simulink model "CCMSensor"  (R2022b)
settings: Solver=FixedStepDiscrete  StartTime=0.0  StopTime=10.0  FixedStep=0.01  RelTol=1e-3
undefined symbols (not defined in the file or in its folder): DioChannelType, IcuChannelType, P_DioLevelType
(part 2 of 2)

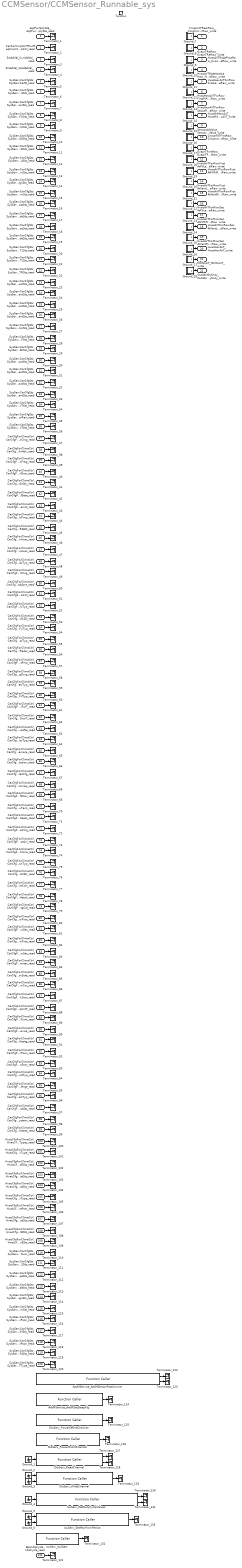
== system CCMSensor/CCMSensor_Runnable_sys ==
  AqsPwrSplySta_AqsPwrSplySta_read : Inport
  FanForCmptmtTRunReqFromClimaCtrl_FanForCmptmtTRunReqFromClimaCtrl_read : Inport  Port=2
  SrsAdVal_CurrAdVal_read : Inport  Port=3
  SrsAdVal_ValidAdVal_read : Inport  Port=4
  SysServCarCfgSts_SysServCarID_read : Inport  Port=5
  SysServCarCfgSts_SysServCCPAQSSts_read : Inport  Port=6
  SysServCarCfgSts_SysServCCPHEXSensorSts_read : Inport  Port=7
  SysServCarCfgSts_SysServCCPLin2AFUSts_read : Inport  Port=8
  SysServCarCfgSts_SysServCCPLin2AIUSts_read : Inport  Port=9
  SysServCarCfgSts_SysServCCPLin2CDSSts_read : Inport  Port=10
  SysServCarCfgSts_SysServCCPLin2PMSISts_read : Inport  Port=11
  SysServCarCfgSts_SysServCCPLin3VGASts_read : Inport  Port=12
  SysServCarCfgSts_SysServDUAirVentSnrSts_read : Inport  Port=13
  SysServCarCfgSts_SysServDUBlowerPwmSignSts_read : Inport  Port=14
  SysServCarCfgSts_SysServDUBlowerPwmSts_read : Inport  Port=15
  SysServCarCfgSts_SysServDUDCDefrostSts_read : Inport  Port=16
  SysServCarCfgSts_SysServDUDCModeSts_read : Inport  Port=17
  SysServCarCfgSts_SysServDUDCRecSts_read : Inport  Port=18
  SysServCarCfgSts_SysServDUDCSModeSts_read : Inport  Port=19
  SysServCarCfgSts_SysServDUDCSTLSts_read : Inport  Port=20
  SysServCarCfgSts_SysServDUDCTLSts_read : Inport  Port=21
  SysServCarCfgSts_SysServDUDCTRSts_read : Inport  Port=22
  SysServCarCfgSts_SysServDUFLFootSts_read : Inport  Port=23
  SysServCarCfgSts_SysServDUFLVentSts_read : Inport  Port=24
  SysServCarCfgSts_SysServDUFRFootSts_read : Inport  Port=25
  SysServCarCfgSts_SysServDUFRVentSts_read : Inport  Port=26
  SysServCarCfgSts_SysServDUIncarMotorSts_read : Inport  Port=27
  SysServCarCfgSts_SysServDULin1HBMFSts_read : Inport  Port=28
  SysServCarCfgSts_SysServDULin4HFAFSts_read : Inport  Port=29
  SysServCarCfgSts_SysServDUSFLFootSts_read : Inport  Port=30
  SysServCarCfgSts_SysServDUSFLVentSts_read : Inport  Port=31
  SysServCarCfgSts_SysServDUSFRFootSts_read : Inport  Port=32
  SysServCarCfgSts_SysServDUSFRVentSts_read : Inport  Port=33
  SysServCarCfgSts_SysServDUSnsrForFCmptmtTSts_read : Inport  Port=34
  SysServCarCfgSts_SysServDUSnsrForRair_read : Inport  Port=35
  SysServCarCfgSts_SysServDUSnsrForRCmptmtTSts_read : Inport  Port=36
  CarCfgForClimaCtrl_CarCfgForAirClng_read : Inport  Port=37
  CarCfgForClimaCtrl_CarCfgForAirClnrAdv_read : Inport  Port=38
  CarCfgForClimaCtrl_CarCfgForAirFrag_read : Inport  Port=39
  CarCfgForClimaCtrl_CarCfgForAirMtrlSnsr_read : Inport  Port=40
  CarCfgForClimaCtrl_CarCfgForAirQly_read : Inport  Port=41
  CarCfgForClimaCtrl_CarCfgForAmntOfSeat_read : Inport  Port=42
  CarCfgForClimaCtrl_CarCfgForAutDemst_read : Inport  Port=43
  CarCfgForClimaCtrl_CarCfgForAuxWtrPmp_read : Inport  Port=44
  CarCfgForClimaCtrl_CarCfgForBRAND_read : Inport  Port=45
  CarCfgForClimaCtrl_CarCfgForBypVlvInHvac_read : Inport  Port=46
  CarCfgForClimaCtrl_CarCfgForClsVlvInHvac_read : Inport  Port=47
  CarCfgForClimaCtrl_CarCfgForCmprAcTyp_read : Inport  Port=48
  CarCfgForClimaCtrl_CarCfgForCmptmtClng_read : Inport  Port=49
  CarCfgForClimaCtrl_CarCfgForElecVentAdjmt_read : Inport  Port=50
  CarCfgForClimaCtrl_CarCfgForExtVentnClimaCtrl_read : Inport  Port=51
  CarCfgForClimaCtrl_CarCfgForFlcTyp_read : Inport  Port=52
  CarCfgForClimaCtrl_CarCfgForFoldCSD_read : Inport  Port=53
  CarCfgForClimaCtrl_CarCfgForFuTyp_read : Inport  Port=54
  CarCfgForClimaCtrl_CarCfgForGlassTyp_read : Inport  Port=55
  CarCfgForClimaCtrl_CarCfgForHeadRadar_read : Inport  Port=56
  CarCfgForClimaCtrl_CarCfgForHeatPmp_read : Inport  Port=57
  CarCfgForClimaCtrl_CarCfgForHeatrDurgDrvg_read : Inport  Port=58
  CarCfgForClimaCtrl_CarCfgForHeatrTyp_read : Inport  Port=59
  CarCfgForClimaCtrl_CarCfgForHvacFilTyp_read : Inport  Port=60
  CarCfgForClimaCtrl_CarCfgForHvacFlrSnsrForT_read : Inport  Port=61
  CarCfgForClimaCtrl_CarCfgForHvacHeatrSnsrT_read : Inport  Port=62
  CarCfgForClimaCtrl_CarCfgForHvacRe_read : Inport  Port=63
  CarCfgForClimaCtrl_CarCfgForHvacTyp_read : Inport  Port=64
  CarCfgForClimaCtrl_CarCfgForInteriorViewCamera_read : Inport  Port=65
  CarCfgForClimaCtrl_CarCfgForLifeDetection_read : Inport  Port=66
  CarCfgForClimaCtrl_CarCfgForLoVoltElecCfg_read : Inport  Port=67
  CarCfgForClimaCtrl_CarCfgForNavSysandIntnmap_read : Inport  Port=68
  CarCfgForClimaCtrl_CarCfgForNrOfDoor_read : Inport  Port=69
  CarCfgForClimaCtrl_CarCfgForPipeInFuTank_read : Inport  Port=70
  CarCfgForClimaCtrl_CarCfgForPowerSeats_read : Inport  Port=71
  CarCfgForClimaCtrl_CarCfgForPreClng_read : Inport  Port=72
  CarCfgForClimaCtrl_CarCfgForPrkgCDSNotAvl_read : Inport  Port=73
  CarCfgForClimaCtrl_CarCfgForPrkgClima_read : Inport  Port=74
  CarCfgForClimaCtrl_CarCfgForPrpsnTyp_read : Inport  Port=75
  CarCfgForClimaCtrl_CarCfgForPTCInFrontHVAC_read : Inport  Port=76
  CarCfgForClimaCtrl_CarCfgForRemEngStrtPreCdn_read : Inport  Port=77
  CarCfgForClimaCtrl_CarCfgForRoofHatch_read : Inport  Port=78
  CarCfgForClimaCtrl_CarCfgForSeatHeatgCal_read : Inport  Port=79
  CarCfgForClimaCtrl_CarCfgForSeatHeatgForRowFirst_read : Inport  Port=80
  CarCfgForClimaCtrl_CarCfgForSeatHeatgForRowSec_read : Inport  Port=81
  CarCfgForClimaCtrl_CarCfgForSeatVentnForRowFirst_read : Inport  Port=82
  CarCfgForClimaCtrl_CarCfgForSeatVentnForRowSec_read : Inport  Port=83
  CarCfgForClimaCtrl_CarCfgForSecRowVentnCenter_read : Inport  Port=84
  CarCfgForClimaCtrl_CarCfgForSecRowVentnSide_read : Inport  Port=85
  CarCfgForClimaCtrl_CarCfgForSmallFlowCyc_read : Inport  Port=86
  CarCfgForClimaCtrl_CarCfgForSmkSnsr_read : Inport  Port=87
  CarCfgForClimaCtrl_CarCfgForSnsrForCmptmtT_read : Inport  Port=88
  CarCfgForClimaCtrl_CarCfgForSnsrHumPrsnt_read : Inport  Port=89
  CarCfgForClimaCtrl_CarCfgForStartDevice_read : Inport  Port=90
  CarCfgForClimaCtrl_CarCfgForSteerWhlHeatg_read : Inport  Port=91
  CarCfgForClimaCtrl_CarCfgForSteerWhlPosn_read : Inport  Port=92
  CarCfgForClimaCtrl_CarCfgForSunSnsr_read : Inport  Port=93
  CarCfgForClimaCtrl_CarCfgForTelmNodTyp_read : Inport  Port=94
  CarCfgForClimaCtrl_CarCfgForThermMngt_read : Inport  Port=95
  CarCfgForClimaCtrl_CarCfgForVehTyp_read : Inport  Port=96
  CarCfgForClimaCtrl_CarCfgForVgaMotorExistSts_read : Inport  Port=97
  CarCfgForClimaCtrl_CarCfgForWadingAssistSystem_read : Inport  Port=98
  CarCfgForClimaCtrl_CarCfgForWindHeatd_read : Inport  Port=99
  HvacCfgForClimaCtrl_HvacCfgForAQSTypes_read : Inport  Port=100
  HvacCfgForClimaCtrl_HvacCfgForHvacFrontBlowerType_read : Inport  Port=101
  HvacCfgForClimaCtrl_HvacCfgForHvacLowSideRETXVESts_read : Inport  Port=102
  HvacCfgForClimaCtrl_HvacCfgForHvacMiscExistSts_read : Inport  Port=103
  HvacCfgForClimaCtrl_HvacCfgForHvacMotorExistSts_read : Inport  Port=104
  HvacCfgForClimaCtrl_HvacCfgForHvacReBlowerType_read : Inport  Port=105
  HvacCfgForClimaCtrl_HvacCfgForHvacReVentPstn_read : Inport  Port=106
  HvacCfgForClimaCtrl_HvacCfgForHvacSensorExistSts_read : Inport  Port=107
  HvacCfgForClimaCtrl_HvacCfgForHvacSnsrRCmptmtESts_read : Inport  Port=108
  HvacCfgForClimaCtrl_HvacCfgForHvacSnsrReHexESts_read : Inport  Port=109
  SysServCarCfgSts_SysServCCPBGMNum_read : Inport  Port=110
  SysServCarCfgSts_SysServCCPLin2AQSSts_read : Inport  Port=111
  SysServCarCfgSts_SysServDUBlowerPwmSignTypeSts_read : Inport  Port=112
  SysServCarCfgSts_SysServDUDCFLMdeSts_read : Inport  Port=113
  SysServCarCfgSts_SysServDUReBlowerPwmSignSts_read : Inport  Port=114
  SysServCarCfgSts_SysServDUReBlowerPwmSts_read : Inport  Port=115
  SysServCarCfgSts_SysServFrntHEXSnsrPosn_read : Inport  Port=116
  SysServCarCfgSts_SysServLowSideRETXVSts_read : Inport  Port=117
  SysServCarCfgSts_SysServReHEXSnsrPosn_read : Inport  Port=118
  SysServCarCfgSts_SysServRHFASts_read : Inport  Port=119
  SysServCarCfgSts_SysServSnsrForFCmptmtTType_read : Inport  Port=120
  BswLifeCycle_LifeCycle_read : Inport  Port=121
  function : TriggerPort  TriggerType=function-call  VariantControl=(inherit)  SampleTimeType=periodic  SampleTime=-1
  AdcMService_AdcMSensorFastConver : FunctionCaller  FunctionPrototype=[SensorFastConverFlag,ERR] = AdcMService_AdcMSensorFastConver()  OutputArgumentSpecifications=P_AdcMSensorFastConver, P_Std_ReturnType
  AdcMService_AdcMSetSleepFlg : FunctionCaller  FunctionPrototype=ERR = AdcMService_AdcMSetSleepFlg()  OutputArgumentSpecifications=P_Std_ReturnType
  DioServ_PowerOffInitChannel : FunctionCaller  FunctionPrototype=ERR = DioServ_PowerOffInitChannel()  OutputArgumentSpecifications=P_Std_ReturnType
  DioServ_PowerOnInitChannel : FunctionCaller  FunctionPrototype=ERR = DioServ_PowerOnInitChannel()  OutputArgumentSpecifications=P_Std_ReturnType
  DioServ_ReadChannel : FunctionCaller  FunctionPrototype=[Level,ERR] = DioServ_ReadChannel(ChannelId)  InputArgumentSpecifications=DioChannelType(0)  OutputArgumentSpecifications=P_DioLevelType, P_Std_ReturnType
  DioServ_WriteChannel : FunctionCaller  FunctionPrototype=ERR = DioServ_WriteChannel(ChannelId,Level)  InputArgumentSpecifications=DioChannelType(0), P_DioLevelType  OutputArgumentSpecifications=P_Std_ReturnType
  Ground_{1..6} (6 alike) : Ground
  Ground_10 : Subsystem
  Ground_11 : Subsystem
  Ground_12 : Subsystem
  Ground_13 : Subsystem
  Ground_14 : Subsystem
  Ground_15 : Subsystem
  Ground_16 : Subsystem
  Ground_17 : Subsystem
  Ground_18 : Subsystem
  Ground_19 : Subsystem
  Ground_20 : Subsystem
  Ground_21 : Subsystem
  Ground_22 : Subsystem
  Ground_23 : Subsystem
  Ground_24 : Subsystem
  Ground_25 : Subsystem
  Ground_26 : Subsystem
  Ground_27 : Subsystem
  Ground_28 : Subsystem
  Ground_7 : Subsystem
  Ground_8 : Subsystem
  Ground_9 : Subsystem
  IcuServ_GetDutyCycleValues : FunctionCaller  FunctionPrototype=[DutyCycleValues,ERR] = IcuServ_GetDutyCycleValues(Channel)  InputArgumentSpecifications=IcuChannelType(0)  OutputArgumentSpecifications=P_IcuDutyCycleType, P_Std_ReturnType
  IcuServ_IcuStart : FunctionCaller  FunctionPrototype=ERR = IcuServ_IcuStart()  OutputArgumentSpecifications=P_Std_ReturnType
  IcuServ_SetMaxPwmPeriod : FunctionCaller  FunctionPrototype=ERR = IcuServ_SetMaxPwmPeriod(Channel,MaxPwmPeriod)  InputArgumentSpecifications=IcuChannelType(0), uint16(1)  OutputArgumentSpecifications=P_Std_ReturnType
  Terminator_{1..133} (133 alike) : Terminator
  CmptmtTRearRaw_CmptmtTRearRaw_write : Outport
      settings: EnsureOutportIsVirtual=on
  EvaprTReRaw_EvaprTReRaw_write : Outport  Port=2
      settings: EnsureOutportIsVirtual=on
  HvacAirTReAtFlrLeRaw_HvacAirTReAtFlrLeRaw_write : Outport  Port=3
      settings: EnsureOutportIsVirtual=on
  HvacAirTReAtVentLeRaw_HvacAirTReAtVentLeRaw_write : Outport  Port=4
      settings: EnsureOutportIsVirtual=on
  HvacHexAirTForRowFirstLeRaw_HvacHexAirTForRowFirstLeRaw_write : Outport  Port=5
      settings: EnsureOutportIsVirtual=on
  HvacHexAirTForRowFirstRiRaw_HvacHexAirTForRowFirstRiRaw_write : Outport  Port=6
      settings: EnsureOutportIsVirtual=on
  HvacHexAirTForRowSecLeRaw_HvacHexAirTForRowSecLeRaw_write : Outport  Port=7
      settings: EnsureOutportIsVirtual=on
  HvacReHexAirT_HvacReHexAirT_write : Outport  Port=8
      settings: EnsureOutportIsVirtual=on
  SensorAdValue_SensorAdValue_write : Outport  Port=9
      settings: EnsureOutportIsVirtual=on
  CmptmtTFrntRaw_CmptmtTFrntRaw_write : Outport  Port=10
      settings: EnsureOutportIsVirtual=on
  EvaprTFrntRaw_EvaprTFrntRaw_write : Outport  Port=11
      settings: EnsureOutportIsVirtual=on
  HvacAirTForRowFirstAtFlrLeRaw_HvacAirTForRowFirstAtFlrLeRaw_write : Outport  Port=12
      settings: EnsureOutportIsVirtual=on
  HvacAirTForRowFirstAtFlrRiRaw_HvacAirTForRowFirstAtFlrRiRaw_write : Outport  Port=13
      settings: EnsureOutportIsVirtual=on
  HvacAirTForRowFirstAtVentLeRaw_HvacAirTForRowFirstAtVentLeRaw_write : Outport  Port=14
      settings: EnsureOutportIsVirtual=on
  HvacAirTForRowFirstAtVentRiRaw_HvacAirTForRowFirstAtVentRiRaw_write : Outport  Port=15
      settings: EnsureOutportIsVirtual=on
  HvacAirTForRowSecAtFlrLeRaw_HvacAirTForRowSecAtFlrLeRaw_write : Outport  Port=16
      settings: EnsureOutportIsVirtual=on
  HvacAirTForRowSecAtFlrRiRaw_HvacAirTForRowSecAtFlrRiRaw_write : Outport  Port=17
      settings: EnsureOutportIsVirtual=on
  HvacAirTForRowSecAtVentLeRaw_HvacAirTForRowSecAtVentLeRaw_write : Outport  Port=18
      settings: EnsureOutportIsVirtual=on
  HvacAirTForRowSecAtVentRiRaw_HvacAirTForRowSecAtVentRiRaw_write : Outport  Port=19
      settings: EnsureOutportIsVirtual=on
  HvacHexAirT_HvacHexAirT_write : Outport  Port=20
      settings: EnsureOutportIsVirtual=on
  MtrlSnsrT_MtrlSnsrT_write : Outport  Port=21
      settings: EnsureOutportIsVirtual=on
  OutdAirQlyDuty_OutdAirQlyDuty_write : Outport  Port=22
      settings: EnsureOutportIsVirtual=on
  wires:
  AqsPwrSplySta_AqsPwrSplySta_read/1 -> Terminator_1/1
  FanForCmptmtTRunReqFromClimaCtrl_FanForCmptmtTRunReqFromClimaCtrl_read/1 -> Terminator_2/1
  SrsAdVal_CurrAdVal_read/1 -> Terminator_3/1
  SrsAdVal_ValidAdVal_read/1 -> Terminator_4/1
  SysServCarCfgSts_SysServCarID_read/1 -> Terminator_5/1
  SysServCarCfgSts_SysServCCPAQSSts_read/1 -> Terminator_6/1
  SysServCarCfgSts_SysServCCPHEXSensorSts_read/1 -> Terminator_7/1
  SysServCarCfgSts_SysServCCPLin2AFUSts_read/1 -> Terminator_8/1
  SysServCarCfgSts_SysServCCPLin2AIUSts_read/1 -> Terminator_9/1
  SysServCarCfgSts_SysServCCPLin2CDSSts_read/1 -> Terminator_10/1
  SysServCarCfgSts_SysServCCPLin2PMSISts_read/1 -> Terminator_11/1
  SysServCarCfgSts_SysServCCPLin3VGASts_read/1 -> Terminator_12/1
  SysServCarCfgSts_SysServDUAirVentSnrSts_read/1 -> Terminator_13/1
  SysServCarCfgSts_SysServDUBlowerPwmSignSts_read/1 -> Terminator_14/1
  SysServCarCfgSts_SysServDUBlowerPwmSts_read/1 -> Terminator_15/1
  SysServCarCfgSts_SysServDUDCDefrostSts_read/1 -> Terminator_16/1
  SysServCarCfgSts_SysServDUDCModeSts_read/1 -> Terminator_17/1
  SysServCarCfgSts_SysServDUDCRecSts_read/1 -> Terminator_18/1
  SysServCarCfgSts_SysServDUDCSModeSts_read/1 -> Terminator_19/1
  SysServCarCfgSts_SysServDUDCSTLSts_read/1 -> Terminator_20/1
  SysServCarCfgSts_SysServDUDCTLSts_read/1 -> Terminator_21/1
  SysServCarCfgSts_SysServDUDCTRSts_read/1 -> Terminator_22/1
  SysServCarCfgSts_SysServDUFLFootSts_read/1 -> Terminator_23/1
  SysServCarCfgSts_SysServDUFLVentSts_read/1 -> Terminator_24/1
  SysServCarCfgSts_SysServDUFRFootSts_read/1 -> Terminator_25/1
  SysServCarCfgSts_SysServDUFRVentSts_read/1 -> Terminator_26/1
  SysServCarCfgSts_SysServDUIncarMotorSts_read/1 -> Terminator_27/1
  SysServCarCfgSts_SysServDULin1HBMFSts_read/1 -> Terminator_28/1
  SysServCarCfgSts_SysServDULin4HFAFSts_read/1 -> Terminator_29/1
  SysServCarCfgSts_SysServDUSFLFootSts_read/1 -> Terminator_30/1
  SysServCarCfgSts_SysServDUSFLVentSts_read/1 -> Terminator_31/1
  SysServCarCfgSts_SysServDUSFRFootSts_read/1 -> Terminator_32/1
  SysServCarCfgSts_SysServDUSFRVentSts_read/1 -> Terminator_33/1
  SysServCarCfgSts_SysServDUSnsrForFCmptmtTSts_read/1 -> Terminator_34/1
  SysServCarCfgSts_SysServDUSnsrForRair_read/1 -> Terminator_35/1
  SysServCarCfgSts_SysServDUSnsrForRCmptmtTSts_read/1 -> Terminator_36/1
  CarCfgForClimaCtrl_CarCfgForAirClng_read/1 -> Terminator_37/1
  CarCfgForClimaCtrl_CarCfgForAirClnrAdv_read/1 -> Terminator_38/1
  CarCfgForClimaCtrl_CarCfgForAirFrag_read/1 -> Terminator_39/1
  CarCfgForClimaCtrl_CarCfgForAirMtrlSnsr_read/1 -> Terminator_40/1
  CarCfgForClimaCtrl_CarCfgForAirQly_read/1 -> Terminator_41/1
  CarCfgForClimaCtrl_CarCfgForAmntOfSeat_read/1 -> Terminator_42/1
  CarCfgForClimaCtrl_CarCfgForAutDemst_read/1 -> Terminator_43/1
  CarCfgForClimaCtrl_CarCfgForAuxWtrPmp_read/1 -> Terminator_44/1
  CarCfgForClimaCtrl_CarCfgForBRAND_read/1 -> Terminator_45/1
  CarCfgForClimaCtrl_CarCfgForBypVlvInHvac_read/1 -> Terminator_46/1
  CarCfgForClimaCtrl_CarCfgForClsVlvInHvac_read/1 -> Terminator_47/1
  CarCfgForClimaCtrl_CarCfgForCmprAcTyp_read/1 -> Terminator_48/1
  CarCfgForClimaCtrl_CarCfgForCmptmtClng_read/1 -> Terminator_49/1
  CarCfgForClimaCtrl_CarCfgForElecVentAdjmt_read/1 -> Terminator_50/1
  CarCfgForClimaCtrl_CarCfgForExtVentnClimaCtrl_read/1 -> Terminator_51/1
  CarCfgForClimaCtrl_CarCfgForFlcTyp_read/1 -> Terminator_52/1
  CarCfgForClimaCtrl_CarCfgForFoldCSD_read/1 -> Terminator_53/1
  CarCfgForClimaCtrl_CarCfgForFuTyp_read/1 -> Terminator_54/1
  CarCfgForClimaCtrl_CarCfgForGlassTyp_read/1 -> Terminator_55/1
  CarCfgForClimaCtrl_CarCfgForHeadRadar_read/1 -> Terminator_56/1
  CarCfgForClimaCtrl_CarCfgForHeatPmp_read/1 -> Terminator_57/1
  CarCfgForClimaCtrl_CarCfgForHeatrDurgDrvg_read/1 -> Terminator_58/1
  CarCfgForClimaCtrl_CarCfgForHeatrTyp_read/1 -> Terminator_59/1
  CarCfgForClimaCtrl_CarCfgForHvacFilTyp_read/1 -> Terminator_60/1
  CarCfgForClimaCtrl_CarCfgForHvacFlrSnsrForT_read/1 -> Terminator_61/1
  CarCfgForClimaCtrl_CarCfgForHvacHeatrSnsrT_read/1 -> Terminator_62/1
  CarCfgForClimaCtrl_CarCfgForHvacRe_read/1 -> Terminator_63/1
  CarCfgForClimaCtrl_CarCfgForHvacTyp_read/1 -> Terminator_64/1
  CarCfgForClimaCtrl_CarCfgForInteriorViewCamera_read/1 -> Terminator_65/1
  CarCfgForClimaCtrl_CarCfgForLifeDetection_read/1 -> Terminator_66/1
  CarCfgForClimaCtrl_CarCfgForLoVoltElecCfg_read/1 -> Terminator_67/1
  CarCfgForClimaCtrl_CarCfgForNavSysandIntnmap_read/1 -> Terminator_68/1
  CarCfgForClimaCtrl_CarCfgForNrOfDoor_read/1 -> Terminator_69/1
  CarCfgForClimaCtrl_CarCfgForPipeInFuTank_read/1 -> Terminator_70/1
  CarCfgForClimaCtrl_CarCfgForPowerSeats_read/1 -> Terminator_71/1
  CarCfgForClimaCtrl_CarCfgForPreClng_read/1 -> Terminator_72/1
  CarCfgForClimaCtrl_CarCfgForPrkgCDSNotAvl_read/1 -> Terminator_73/1
  CarCfgForClimaCtrl_CarCfgForPrkgClima_read/1 -> Terminator_74/1
  CarCfgForClimaCtrl_CarCfgForPrpsnTyp_read/1 -> Terminator_75/1
  CarCfgForClimaCtrl_CarCfgForPTCInFrontHVAC_read/1 -> Terminator_76/1
  CarCfgForClimaCtrl_CarCfgForRemEngStrtPreCdn_read/1 -> Terminator_77/1
  CarCfgForClimaCtrl_CarCfgForRoofHatch_read/1 -> Terminator_78/1
  CarCfgForClimaCtrl_CarCfgForSeatHeatgCal_read/1 -> Terminator_79/1
  CarCfgForClimaCtrl_CarCfgForSeatHeatgForRowFirst_read/1 -> Terminator_80/1
  CarCfgForClimaCtrl_CarCfgForSeatHeatgForRowSec_read/1 -> Terminator_81/1
  CarCfgForClimaCtrl_CarCfgForSeatVentnForRowFirst_read/1 -> Terminator_82/1
  CarCfgForClimaCtrl_CarCfgForSeatVentnForRowSec_read/1 -> Terminator_83/1
  CarCfgForClimaCtrl_CarCfgForSecRowVentnCenter_read/1 -> Terminator_84/1
  CarCfgForClimaCtrl_CarCfgForSecRowVentnSide_read/1 -> Terminator_85/1
  CarCfgForClimaCtrl_CarCfgForSmallFlowCyc_read/1 -> Terminator_86/1
  CarCfgForClimaCtrl_CarCfgForSmkSnsr_read/1 -> Terminator_87/1
  CarCfgForClimaCtrl_CarCfgForSnsrForCmptmtT_read/1 -> Terminator_88/1
  CarCfgForClimaCtrl_CarCfgForSnsrHumPrsnt_read/1 -> Terminator_89/1
  CarCfgForClimaCtrl_CarCfgForStartDevice_read/1 -> Terminator_90/1
  CarCfgForClimaCtrl_CarCfgForSteerWhlHeatg_read/1 -> Terminator_91/1
  CarCfgForClimaCtrl_CarCfgForSteerWhlPosn_read/1 -> Terminator_92/1
  CarCfgForClimaCtrl_CarCfgForSunSnsr_read/1 -> Terminator_93/1
  CarCfgForClimaCtrl_CarCfgForTelmNodTyp_read/1 -> Terminator_94/1
  CarCfgForClimaCtrl_CarCfgForThermMngt_read/1 -> Terminator_95/1
  CarCfgForClimaCtrl_CarCfgForVehTyp_read/1 -> Terminator_96/1
  CarCfgForClimaCtrl_CarCfgForVgaMotorExistSts_read/1 -> Terminator_97/1
  CarCfgForClimaCtrl_CarCfgForWadingAssistSystem_read/1 -> Terminator_98/1
  CarCfgForClimaCtrl_CarCfgForWindHeatd_read/1 -> Terminator_99/1
  HvacCfgForClimaCtrl_HvacCfgForAQSTypes_read/1 -> Terminator_100/1
  HvacCfgForClimaCtrl_HvacCfgForHvacFrontBlowerType_read/1 -> Terminator_101/1
  HvacCfgForClimaCtrl_HvacCfgForHvacLowSideRETXVESts_read/1 -> Terminator_102/1
  HvacCfgForClimaCtrl_HvacCfgForHvacMiscExistSts_read/1 -> Terminator_103/1
  HvacCfgForClimaCtrl_HvacCfgForHvacMotorExistSts_read/1 -> Terminator_104/1
  HvacCfgForClimaCtrl_HvacCfgForHvacReBlowerType_read/1 -> Terminator_105/1
  HvacCfgForClimaCtrl_HvacCfgForHvacReVentPstn_read/1 -> Terminator_106/1
  HvacCfgForClimaCtrl_HvacCfgForHvacSensorExistSts_read/1 -> Terminator_107/1
  HvacCfgForClimaCtrl_HvacCfgForHvacSnsrRCmptmtESts_read/1 -> Terminator_108/1
  HvacCfgForClimaCtrl_HvacCfgForHvacSnsrReHexESts_read/1 -> Terminator_109/1
  SysServCarCfgSts_SysServCCPBGMNum_read/1 -> Terminator_110/1
  SysServCarCfgSts_SysServCCPLin2AQSSts_read/1 -> Terminator_111/1
  SysServCarCfgSts_SysServDUBlowerPwmSignTypeSts_read/1 -> Terminator_112/1
  SysServCarCfgSts_SysServDUDCFLMdeSts_read/1 -> Terminator_113/1
  SysServCarCfgSts_SysServDUReBlowerPwmSignSts_read/1 -> Terminator_114/1
  SysServCarCfgSts_SysServDUReBlowerPwmSts_read/1 -> Terminator_115/1
  SysServCarCfgSts_SysServFrntHEXSnsrPosn_read/1 -> Terminator_116/1
  SysServCarCfgSts_SysServLowSideRETXVSts_read/1 -> Terminator_117/1
  SysServCarCfgSts_SysServReHEXSnsrPosn_read/1 -> Terminator_118/1
  SysServCarCfgSts_SysServRHFASts_read/1 -> Terminator_119/1
  SysServCarCfgSts_SysServSnsrForFCmptmtTType_read/1 -> Terminator_120/1
  BswLifeCycle_LifeCycle_read/1 -> Terminator_121/1
  AdcMService_AdcMSensorFastConver/1 -> Terminator_122/1
  AdcMService_AdcMSensorFastConver/2 -> Terminator_123/1
  AdcMService_AdcMSetSleepFlg/1 -> Terminator_124/1
  DioServ_PowerOffInitChannel/1 -> Terminator_125/1
  DioServ_PowerOnInitChannel/1 -> Terminator_126/1
  Ground_1/1 -> DioServ_ReadChannel/1
  DioServ_ReadChannel/1 -> Terminator_127/1
  DioServ_ReadChannel/2 -> Terminator_128/1
  Ground_2/1 -> DioServ_WriteChannel/1
  Ground_3/1 -> DioServ_WriteChannel/2
  DioServ_WriteChannel/1 -> Terminator_129/1
  Ground_4/1 -> IcuServ_GetDutyCycleValues/1
  IcuServ_GetDutyCycleValues/1 -> Terminator_130/1
  IcuServ_GetDutyCycleValues/2 -> Terminator_131/1
  IcuServ_IcuStart/1 -> Terminator_132/1
  Ground_5/1 -> IcuServ_SetMaxPwmPeriod/1
  Ground_6/1 -> IcuServ_SetMaxPwmPeriod/2
  IcuServ_SetMaxPwmPeriod/1 -> Terminator_133/1
  Ground_7/1(Out1) -> CmptmtTRearRaw_CmptmtTRearRaw_write/1
  Ground_8/1(Out1) -> EvaprTReRaw_EvaprTReRaw_write/1
  Ground_9/1(Out1) -> HvacAirTReAtFlrLeRaw_HvacAirTReAtFlrLeRaw_write/1
  Ground_10/1(Out1) -> HvacAirTReAtVentLeRaw_HvacAirTReAtVentLeRaw_write/1
  Ground_11/1(Out1) -> HvacHexAirTForRowFirstLeRaw_HvacHexAirTForRowFirstLeRaw_write/1
  Ground_12/1(Out1) -> HvacHexAirTForRowFirstRiRaw_HvacHexAirTForRowFirstRiRaw_write/1
  Ground_13/1(Out1) -> HvacHexAirTForRowSecLeRaw_HvacHexAirTForRowSecLeRaw_write/1
  Ground_14/1(Out1) -> HvacReHexAirT_HvacReHexAirT_write/1
  Ground_15/1(Out1) -> SensorAdValue_SensorAdValue_write/1
  Ground_16/1(Out1) -> CmptmtTFrntRaw_CmptmtTFrntRaw_write/1
  Ground_17/1(Out1) -> EvaprTFrntRaw_EvaprTFrntRaw_write/1
  Ground_18/1(Out1) -> HvacAirTForRowFirstAtFlrLeRaw_HvacAirTForRowFirstAtFlrLeRaw_write/1
  Ground_19/1(Out1) -> HvacAirTForRowFirstAtFlrRiRaw_HvacAirTForRowFirstAtFlrRiRaw_write/1
  Ground_20/1(Out1) -> HvacAirTForRowFirstAtVentLeRaw_HvacAirTForRowFirstAtVentLeRaw_write/1
  Ground_21/1(Out1) -> HvacAirTForRowFirstAtVentRiRaw_HvacAirTForRowFirstAtVentRiRaw_write/1
  Ground_22/1(Out1) -> HvacAirTForRowSecAtFlrLeRaw_HvacAirTForRowSecAtFlrLeRaw_write/1
  Ground_23/1(Out1) -> HvacAirTForRowSecAtFlrRiRaw_HvacAirTForRowSecAtFlrRiRaw_write/1
  Ground_24/1(Out1) -> HvacAirTForRowSecAtVentLeRaw_HvacAirTForRowSecAtVentLeRaw_write/1
  Ground_25/1(Out1) -> HvacAirTForRowSecAtVentRiRaw_HvacAirTForRowSecAtVentRiRaw_write/1
  Ground_26/1(Out1) -> HvacHexAirT_HvacHexAirT_write/1
  Ground_27/1(Out1) -> MtrlSnsrT_MtrlSnsrT_write/1
  Ground_28/1(Out1) -> OutdAirQlyDuty_OutdAirQlyDuty_write/1

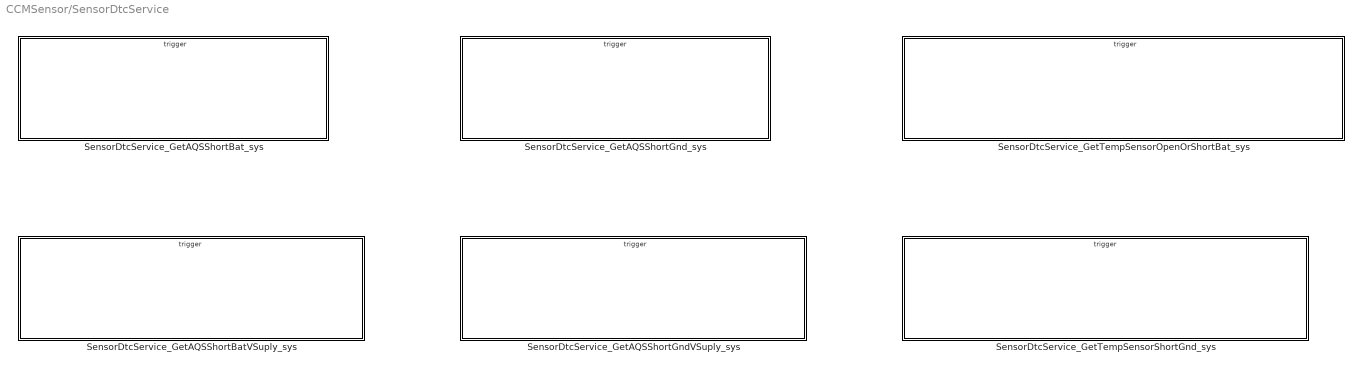
== system CCMSensor/SensorDtcService ==
  SensorDtcService_GetAQSShortBatVSuply_sys : Subsystem
  SensorDtcService_GetAQSShortBat_sys : Subsystem
  SensorDtcService_GetAQSShortGndVSuply_sys : Subsystem
  SensorDtcService_GetAQSShortGnd_sys : Subsystem
  SensorDtcService_GetTempSensorOpenOrShortBat_sys : Subsystem
  SensorDtcService_GetTempSensorShortGnd_sys : Subsystem

== system CCMSensor/SensorParaService ==
  SensorParaService_GetValidValue_sys : Subsystem

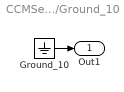
== system CCMSensor/CCMSensor_Runnable_sys/Ground_10 ==
  Ground_10 : Ground
  Out1 : Outport  OutDataTypeStr=Bus: HvacAirTReAtVentLe  PortDimensions=1
  wires:
  Ground_10/1 -> Out1/1

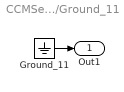
== system CCMSensor/CCMSensor_Runnable_sys/Ground_11 ==
  Ground_11 : Ground
  Out1 : Outport  OutDataTypeStr=Bus: HvacHexAirTForRowFirstLe  PortDimensions=1
  wires:
  Ground_11/1 -> Out1/1

== system CCMSensor/CCMSensor_Runnable_sys/Ground_12 ==
  Ground_12 : Ground
  Out1 : Outport  OutDataTypeStr=Bus: HvacHexAirTForRowFirstRi  PortDimensions=1
  wires:
  Ground_12/1 -> Out1/1

== system CCMSensor/CCMSensor_Runnable_sys/Ground_13 ==
  Ground_13 : Ground
  Out1 : Outport  OutDataTypeStr=Bus: HvacHexAirTForRowSecLe  PortDimensions=1
  wires:
  Ground_13/1 -> Out1/1

== system CCMSensor/CCMSensor_Runnable_sys/Ground_14 ==
  Ground_14 : Ground
  Out1 : Outport  OutDataTypeStr=Bus: HvacAirTForHeatrFrnt  PortDimensions=1
  wires:
  Ground_14/1 -> Out1/1

== system CCMSensor/CCMSensor_Runnable_sys/Ground_15 ==
  Ground_15 : Ground
  Out1 : Outport  OutDataTypeStr=Bus: SensorAd  PortDimensions=1
  wires:
  Ground_15/1 -> Out1/1

== system CCMSensor/CCMSensor_Runnable_sys/Ground_16 ==
  Ground_16 : Ground
  Out1 : Outport  OutDataTypeStr=Bus: CmptmtTFrnt  PortDimensions=1
  wires:
  Ground_16/1 -> Out1/1

== system CCMSensor/CCMSensor_Runnable_sys/Ground_17 ==
  Ground_17 : Ground
  Out1 : Outport  OutDataTypeStr=Bus: EvaprTFrnt  PortDimensions=1
  wires:
  Ground_17/1 -> Out1/1

== system CCMSensor/CCMSensor_Runnable_sys/Ground_18 ==
  Ground_18 : Ground
  Out1 : Outport  OutDataTypeStr=Bus: HvacAirTForRowFirstAtFlrLe  PortDimensions=1
  wires:
  Ground_18/1 -> Out1/1

== system CCMSensor/CCMSensor_Runnable_sys/Ground_19 ==
  Ground_19 : Ground
  Out1 : Outport  OutDataTypeStr=Bus: HvacAirTForRowFirstAtFlrRi  PortDimensions=1
  wires:
  Ground_19/1 -> Out1/1

== system CCMSensor/CCMSensor_Runnable_sys/Ground_20 ==
  Ground_20 : Ground
  Out1 : Outport  OutDataTypeStr=Bus: HvacAirTForRowFirstAtVentLe  PortDimensions=1
  wires:
  Ground_20/1 -> Out1/1

== system CCMSensor/CCMSensor_Runnable_sys/Ground_21 ==
  Ground_21 : Ground
  Out1 : Outport  OutDataTypeStr=Bus: HvacAirTForRowFirstAtVentRi  PortDimensions=1
  wires:
  Ground_21/1 -> Out1/1

== system CCMSensor/CCMSensor_Runnable_sys/Ground_22 ==
  Ground_22 : Ground
  Out1 : Outport  OutDataTypeStr=Bus: HvacAirTForRowSecAtFlrLe  PortDimensions=1
  wires:
  Ground_22/1 -> Out1/1

== system CCMSensor/CCMSensor_Runnable_sys/Ground_23 ==
  Ground_23 : Ground
  Out1 : Outport  OutDataTypeStr=Bus: HvacAirTForRowSecAtFlrRi  PortDimensions=1
  wires:
  Ground_23/1 -> Out1/1

== system CCMSensor/CCMSensor_Runnable_sys/Ground_24 ==
  Ground_24 : Ground
  Out1 : Outport  OutDataTypeStr=Bus: HvacAirTForRowSecAtVentLe  PortDimensions=1
  wires:
  Ground_24/1 -> Out1/1

== system CCMSensor/CCMSensor_Runnable_sys/Ground_25 ==
  Ground_25 : Ground
  Out1 : Outport  OutDataTypeStr=Bus: HvacAirTForRowSecAtVentRi  PortDimensions=1
  wires:
  Ground_25/1 -> Out1/1

== system CCMSensor/CCMSensor_Runnable_sys/Ground_26 ==
  Ground_26 : Ground
  Out1 : Outport  OutDataTypeStr=Bus: HvacAirTForHeatrFrnt  PortDimensions=1
  wires:
  Ground_26/1 -> Out1/1

== system CCMSensor/CCMSensor_Runnable_sys/Ground_27 ==
  Ground_27 : Ground
  Out1 : Outport  OutDataTypeStr=Bus: MtrlSnsrT  PortDimensions=1
  wires:
  Ground_27/1 -> Out1/1

== system CCMSensor/CCMSensor_Runnable_sys/Ground_28 ==
  Ground_28 : Ground
  Out1 : Outport  OutDataTypeStr=Bus: OutdAirQlyDutyWithQly  PortDimensions=1
  wires:
  Ground_28/1 -> Out1/1

== system CCMSensor/CCMSensor_Runnable_sys/Ground_7 ==
  Ground_7 : Ground
  Out1 : Outport  OutDataTypeStr=Bus: CmptmtTFrnt  PortDimensions=1
  wires:
  Ground_7/1 -> Out1/1

== system CCMSensor/CCMSensor_Runnable_sys/Ground_8 ==
  Ground_8 : Ground
  Out1 : Outport  OutDataTypeStr=Bus: EvaprTFrnt  PortDimensions=1
  wires:
  Ground_8/1 -> Out1/1

== system CCMSensor/CCMSensor_Runnable_sys/Ground_9 ==
  Ground_9 : Ground
  Out1 : Outport  OutDataTypeStr=Bus: HvacAirTReAtFlrLe  PortDimensions=1
  wires:
  Ground_9/1 -> Out1/1

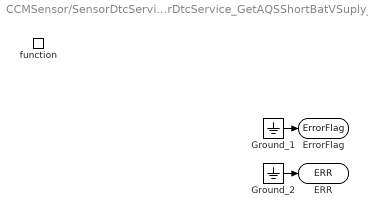
== system CCMSensor/SensorDtcService/SensorDtcService_GetAQSShortBatVSuply_sys ==
  function : TriggerPort  TriggerType=function-call  IsSimulinkFunction=on  FunctionName=SensorDtcService_GetAQSShortBatVSuply  VariantControl=(inherit)
  Ground_1 : Ground
  Ground_2 : Ground
  ErrorFlag : ArgOut  OutDataTypeStr=Enum: DTCState  ArgumentName=ErrorFlag
  ERR : ArgOut  Port=2  OutDataTypeStr=Std_ReturnType  ArgumentName=ERR
  wires:
  Ground_1/1 -> ErrorFlag/1
  Ground_2/1 -> ERR/1

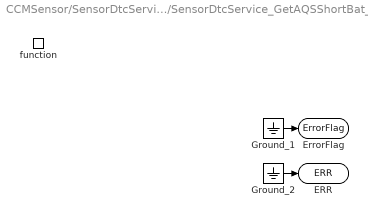
== system CCMSensor/SensorDtcService/SensorDtcService_GetAQSShortBat_sys ==
  function : TriggerPort  TriggerType=function-call  IsSimulinkFunction=on  FunctionName=SensorDtcService_GetAQSShortBat  VariantControl=(inherit)
  Ground_1 : Ground
  Ground_2 : Ground
  ErrorFlag : ArgOut  OutDataTypeStr=Enum: DTCState  ArgumentName=ErrorFlag
  ERR : ArgOut  Port=2  OutDataTypeStr=Std_ReturnType  ArgumentName=ERR
  wires:
  Ground_1/1 -> ErrorFlag/1
  Ground_2/1 -> ERR/1

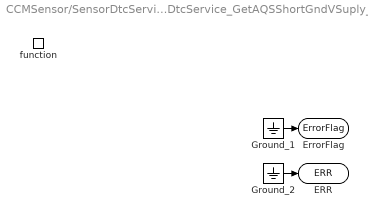
== system CCMSensor/SensorDtcService/SensorDtcService_GetAQSShortGndVSuply_sys ==
  function : TriggerPort  TriggerType=function-call  IsSimulinkFunction=on  FunctionName=SensorDtcService_GetAQSShortGndVSuply  VariantControl=(inherit)
  Ground_1 : Ground
  Ground_2 : Ground
  ErrorFlag : ArgOut  OutDataTypeStr=Enum: DTCState  ArgumentName=ErrorFlag
  ERR : ArgOut  Port=2  OutDataTypeStr=Std_ReturnType  ArgumentName=ERR
  wires:
  Ground_1/1 -> ErrorFlag/1
  Ground_2/1 -> ERR/1

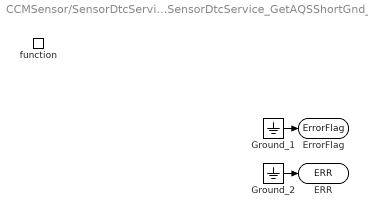
== system CCMSensor/SensorDtcService/SensorDtcService_GetAQSShortGnd_sys ==
  function : TriggerPort  TriggerType=function-call  IsSimulinkFunction=on  FunctionName=SensorDtcService_GetAQSShortGnd  VariantControl=(inherit)
  Ground_1 : Ground
  Ground_2 : Ground
  ErrorFlag : ArgOut  OutDataTypeStr=Enum: DTCState  ArgumentName=ErrorFlag
  ERR : ArgOut  Port=2  OutDataTypeStr=Std_ReturnType  ArgumentName=ERR
  wires:
  Ground_1/1 -> ErrorFlag/1
  Ground_2/1 -> ERR/1

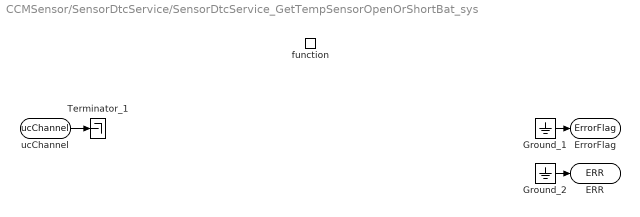
== system CCMSensor/SensorDtcService/SensorDtcService_GetTempSensorOpenOrShortBat_sys ==
  function : TriggerPort  TriggerType=function-call  IsSimulinkFunction=on  FunctionName=SensorDtcService_GetTempSensorOpenOrShortBat  VariantControl=(inherit)
  Ground_1 : Ground
  Ground_2 : Ground
  Terminator_1 : Terminator
  ucChannel : ArgIn  OutDataTypeStr=Enum: SrsAdChlType  ArgumentName=ucChannel
  ErrorFlag : ArgOut  OutDataTypeStr=Enum: DTCState  ArgumentName=ErrorFlag
  ERR : ArgOut  Port=2  OutDataTypeStr=Std_ReturnType  ArgumentName=ERR
  wires:
  ucChannel/1 -> Terminator_1/1
  Ground_1/1 -> ErrorFlag/1
  Ground_2/1 -> ERR/1

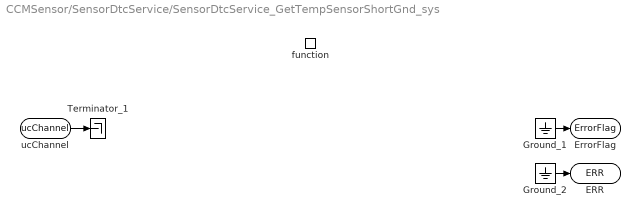
== system CCMSensor/SensorDtcService/SensorDtcService_GetTempSensorShortGnd_sys ==
  function : TriggerPort  TriggerType=function-call  IsSimulinkFunction=on  FunctionName=SensorDtcService_GetTempSensorShortGnd  VariantControl=(inherit)
  Ground_1 : Ground
  Ground_2 : Ground
  Terminator_1 : Terminator
  ucChannel : ArgIn  OutDataTypeStr=Enum: SrsAdChlType  ArgumentName=ucChannel
  ErrorFlag : ArgOut  OutDataTypeStr=Enum: DTCState  ArgumentName=ErrorFlag
  ERR : ArgOut  Port=2  OutDataTypeStr=Std_ReturnType  ArgumentName=ERR
  wires:
  ucChannel/1 -> Terminator_1/1
  Ground_1/1 -> ErrorFlag/1
  Ground_2/1 -> ERR/1

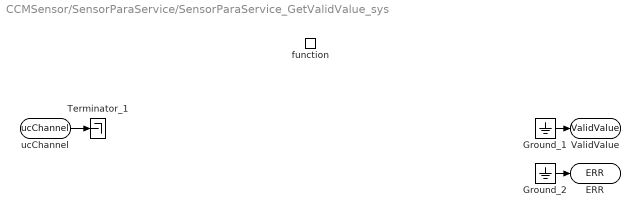
== system CCMSensor/SensorParaService/SensorParaService_GetValidValue_sys ==
  function : TriggerPort  TriggerType=function-call  IsSimulinkFunction=on  FunctionName=SensorParaService_GetValidValue  VariantControl=(inherit)
  Ground_1 : Ground
  Ground_2 : Ground
  Terminator_1 : Terminator
  ucChannel : ArgIn  OutDataTypeStr=Enum: SrsAdChlType  ArgumentName=ucChannel
  ValidValue : ArgOut  OutDataTypeStr=UInt16  ArgumentName=ValidValue
  ERR : ArgOut  Port=2  OutDataTypeStr=Std_ReturnType  ArgumentName=ERR
  wires:
  ucChannel/1 -> Terminator_1/1
  Ground_1/1 -> ValidValue/1
  Ground_2/1 -> ERR/1
